simulink model "MX_Gateway"  (Simulink 9.0)
settings: Solver=FixedStepDiscrete  StartTime=0.0  StopTime=100  FixedStep=0.01  RelTol=1e-3
values from neighbouring files (symbols this model uses):
  ACTIVE = Bobcat.Parameter; CreateDefine( ACTIVE                         ,1                          ,'Define' ,'defines.h' )    [defines.m]
  ACTIVE_MODE_ONE = Bobcat.Parameter; CreateDefine( ACTIVE_MODE_ONE                ,19                         ,'Define' ,'defines.h' )    [defines.m]
  AUTHENTICATED = Bobcat.Parameter; CreateDefine( AUTHENTICATED                  ,1                          ,'Define' ,'defines.h' )    [defines.m]
  AUX_EMEA_CONTROLS = Bobcat.Parameter; CreateDefine( AUX_EMEA_CONTROLS  ,1   ,'Define' ,'defines.h' )    [enums.m]
  AUX_FLOW_HIGH = Bobcat.Parameter; CreateDefine( AUX_FLOW_HIGH           ,4  ,'Define' ,'defines.h' )    [enums.m]
  AUX_FLOW_INACTIVE = Bobcat.Parameter; CreateDefine( AUX_FLOW_INACTIVE       ,0  ,'Define' ,'defines.h' )    [enums.m]
  AUX_FLOW_LOW = Bobcat.Parameter; CreateDefine( AUX_FLOW_LOW            ,1  ,'Define' ,'defines.h' )    [enums.m]
  AUX_FLOW_MEDIUM = Bobcat.Parameter; CreateDefine( AUX_FLOW_MEDIUM         ,2  ,'Define' ,'defines.h' )    [enums.m]
  AUX_FLOW_STANDARD = Bobcat.Parameter; CreateDefine( AUX_FLOW_STANDARD       ,3  ,'Define' ,'defines.h' )    [enums.m]
  AUX_NON_FUNCTIONAL = Bobcat.Parameter; CreateDefine( AUX_NON_FUNCTIONAL ,255 ,'Define' ,'defines.h' )    [enums.m]
  BASE_ACTIVE = Bobcat.Parameter; CreateDefine( BASE_ACTIVE                    ,253                        ,'Define' ,'defines.h' )    [defines.m]
  BASE_DETENT = Bobcat.Parameter; CreateDefine( BASE_DETENT                    ,1                          ,'Define' ,'defines.h' )    [defines.m]
  BEEP_NONE = Bobcat.Parameter; CreateDefine( BEEP_NONE                      ,255                        ,'Define' ,'defines.h' )    [defines.m]
  CD_AIR_FILTER_DEBOUNCE_NORMAL = Bobcat.Parameter; CreateAliasCD( CD_AIR_FILTER_DEBOUNCE_NORMAL                        ,uint16(500)                ,'ConstantDataPointer' ,'CONSTANT_DATA' ,'AIR_FILTER_DEBOUNCE_NORMAL')    [cdata.m]    (also in hc12_cdata.m; m4_cdata.m)
  CD_AIR_FILTER_DEBOUNCE_NOT_CONNECTED = Bobcat.Parameter; CreateAliasCD( CD_AIR_FILTER_DEBOUNCE_NOT_CONNECTED                 ,uint16(3000)               ,'ConstantDataPointer' ,'CONSTANT_DATA' ,'AIR_FILTER_DEBOUNCE_NOT_CONNECTED')    [cdata.m]    (also in hc12_cdata.m; m4_cdata.m)
  CD_AIR_FILTER_DEBOUNCE_PLUGGED = Bobcat.Parameter; CreateAliasCD( CD_AIR_FILTER_DEBOUNCE_PLUGGED                       ,uint16(3000)               ,'ConstantDataPointer' ,'CONSTANT_DATA' ,'AIR_FILTER_DEBOUNCE_PLUGGED')    [cdata.m]    (also in hc12_cdata.m; m4_cdata.m)
  CD_AIR_FILTER_FUNCTION = Bobcat.Parameter; CreateAliasCD( CD_AIR_FILTER_FUNCTION                               ,uint8(255)                 ,'ConstantDataPointer' ,'CONSTANT_DATA' ,'AIR_FILTER_FUNCTION')    [cdata.m]    (also in hc12_cdata.m; m4_cdata.m)
  CD_AIR_FILTER_PLUGGED_WEIGHT = Bobcat.Parameter; CreateAliasCD( CD_AIR_FILTER_PLUGGED_WEIGHT                         ,uint8(2)                   ,'ConstantDataPointer' ,'CONSTANT_DATA' ,'AIR_FILTER_PLUGGED_WEIGHT')    [cdata.m]    (also in hc12_cdata.m; m4_cdata.m)
  CD_AIR_FILTER_UNPLUGGED_WEIGHT = Bobcat.Parameter; CreateAliasCD( CD_AIR_FILTER_UNPLUGGED_WEIGHT                       ,uint8(1)                   ,'ConstantDataPointer' ,'CONSTANT_DATA' ,'AIR_FILTER_UNPLUGGED_WEIGHT')    [cdata.m]    (also in hc12_cdata.m; m4_cdata.m)
  CD_AUTOIDLE_ARMBAR_DELAY = Bobcat.Parameter; CreateAliasCD( CD_AUTOIDLE_ARMBAR_DELAY                             ,uint8(2)                   ,'ConstantDataPointer' ,'CONSTANT_DATA' ,'AUTOIDLE_ARMBAR_DELAY')    [cdata.m]    (also in hc12_cdata.m; m4_cdata.m)
  CD_AUTOIDLE_FUNCTION = Bobcat.Parameter; CreateAliasCD( CD_AUTOIDLE_FUNCTION                                 ,uint8(255)                 ,'ConstantDataPointer' ,'CONSTANT_DATA' ,'AUTOIDLE_FUNCTION')    [cdata.m]    (also in hc12_cdata.m; m4_cdata.m)
  CD_AUTOIDLE_IDLE_DELAY = Bobcat.Parameter; CreateAliasCD( CD_AUTOIDLE_IDLE_DELAY                               ,uint8(40)                  ,'ConstantDataPointer' ,'CONSTANT_DATA' ,'AUTOIDLE_IDLE_DELAY')    [cdata.m]    (also in hc12_cdata.m; m4_cdata.m)
  CD_AUTOIDLE_LOW_IDLE_START = Bobcat.Parameter; CreateAliasCD( CD_AUTOIDLE_LOW_IDLE_START                           ,uint8(255)                 ,'ConstantDataPointer' ,'CONSTANT_DATA' ,'AUTOIDLE_LOW_IDLE_START')    [cdata.m]    (also in hc12_cdata.m; m4_cdata.m)
  CD_AUTOIDLE_PRESSURE_OFF = Bobcat.Parameter; CreateAliasCD( CD_AUTOIDLE_PRESSURE_OFF                             ,uint16(300)                ,'ConstantDataPointer' ,'CONSTANT_DATA' ,'AUTOIDLE_PRESSURE_OFF')    [cdata.m]    (also in hc12_cdata.m; m4_cdata.m)
  CD_AUTOIDLE_PRESSURE_ON = Bobcat.Parameter; CreateAliasCD( CD_AUTOIDLE_PRESSURE_ON                              ,uint16(270)                ,'ConstantDataPointer' ,'CONSTANT_DATA' ,'AUTOIDLE_PRESSURE_ON')    [cdata.m]    (also in hc12_cdata.m; m4_cdata.m)
  CD_AUTOSHIFT_FUNCTION = Bobcat.Parameter; CreateAliasCD( CD_AUTOSHIFT_FUNCTION                                ,uint8(255)                 ,'ConstantDataPointer' ,'CONSTANT_DATA' ,'AUTOSHIFT_FUNCTION')    [cdata.m]    (also in hc12_cdata.m; m4_cdata.m)
  CD_AUTOSHIFT_PHIGH1 = Bobcat.Parameter; CreateAliasCD( CD_AUTOSHIFT_PHIGH1                                  ,uint16(205)                ,'ConstantDataPointer' ,'CONSTANT_DATA' ,'AUTOSHIFT_PHIGH1')    [cdata.m]    (also in hc12_cdata.m; m4_cdata.m)
  CD_AUTOSHIFT_PHIGH2 = Bobcat.Parameter; CreateAliasCD( CD_AUTOSHIFT_PHIGH2                                  ,uint16(193)                ,'ConstantDataPointer' ,'CONSTANT_DATA' ,'AUTOSHIFT_PHIGH2')    [cdata.m]    (also in hc12_cdata.m; m4_cdata.m)
  CD_AUTOSHIFT_PHIGH_TEMP1 = Bobcat.Parameter; CreateAliasCD( CD_AUTOSHIFT_PHIGH_TEMP1                             ,int16(70)                  ,'ConstantDataPointer' ,'CONSTANT_DATA' ,'AUTOSHIFT_PHIGH_TEMP1')    [cdata.m]    (also in hc12_cdata.m; m4_cdata.m)
  CD_AUTOSHIFT_PHIGH_TEMP2 = Bobcat.Parameter; CreateAliasCD( CD_AUTOSHIFT_PHIGH_TEMP2                             ,int16(160)                 ,'ConstantDataPointer' ,'CONSTANT_DATA' ,'AUTOSHIFT_PHIGH_TEMP2')    [cdata.m]    (also in hc12_cdata.m; m4_cdata.m)
  CD_AUTOSHIFT_PLOW1 = Bobcat.Parameter; CreateAliasCD( CD_AUTOSHIFT_PLOW1                                   ,uint16(130)                ,'ConstantDataPointer' ,'CONSTANT_DATA' ,'AUTOSHIFT_PLOW1')    [cdata.m]    (also in hc12_cdata.m; m4_cdata.m)
  CD_AUTOSHIFT_PLOW2 = Bobcat.Parameter; CreateAliasCD( CD_AUTOSHIFT_PLOW2                                   ,uint16(100)                ,'ConstantDataPointer' ,'CONSTANT_DATA' ,'AUTOSHIFT_PLOW2')    [cdata.m]    (also in hc12_cdata.m; m4_cdata.m)
  CD_AUTOSHIFT_PLOW_TEMP1 = Bobcat.Parameter; CreateAliasCD( CD_AUTOSHIFT_PLOW_TEMP1                              ,int16(20)                  ,'ConstantDataPointer' ,'CONSTANT_DATA' ,'AUTOSHIFT_PLOW_TEMP1')    [cdata.m]    (also in hc12_cdata.m; m4_cdata.m)
  CD_AUTOSHIFT_PLOW_TEMP2 = Bobcat.Parameter; CreateAliasCD( CD_AUTOSHIFT_PLOW_TEMP2                              ,int16(80)                  ,'ConstantDataPointer' ,'CONSTANT_DATA' ,'AUTOSHIFT_PLOW_TEMP2')    [cdata.m]    (also in hc12_cdata.m; m4_cdata.m)
  CD_AUTOSHIFT_TRAVEL_DELAY = Bobcat.Parameter; CreateAliasCD( CD_AUTOSHIFT_TRAVEL_DELAY                            ,uint8(32)                  ,'ConstantDataPointer' ,'CONSTANT_DATA' ,'AUTOSHIFT_TRAVEL_DELAY')    [cdata.m]    (also in hc12_cdata.m; m4_cdata.m)
  CD_AUX2_CRACKPOINT_CURRENT = Bobcat.Parameter; CreateAliasCD( CD_AUX2_CRACKPOINT_CURRENT                           ,fi(0,0,16,12,'hex','0c28') ,'ConstantDataPointer' ,'CONSTANT_DATA' ,'AUX2_CRACKPOINT_CURRENT')    [cdata.m]    (also in hc12_cdata.m; m4_cdata.m)
  CD_AUX2_FLOW_LOW_FUNCTION = Bobcat.Parameter; CreateAliasCD( CD_AUX2_FLOW_LOW_FUNCTION                            ,uint8(255)                 ,'ConstantDataPointer' ,'CONSTANT_DATA' ,'AUX2_FLOW_LOW_FUNCTION')    [cdata.m]    (also in hc12_cdata.m; m4_cdata.m)
  CD_AUX2_FLOW_MED_FUNCTION = Bobcat.Parameter; CreateAliasCD( CD_AUX2_FLOW_MED_FUNCTION                            ,uint8(255)                 ,'ConstantDataPointer' ,'CONSTANT_DATA' ,'AUX2_FLOW_MED_FUNCTION')    [cdata.m]    (also in hc12_cdata.m; m4_cdata.m)
  CD_AUX2_HI_FLOW_PERCENT = Bobcat.Parameter; CreateAliasCD( CD_AUX2_HI_FLOW_PERCENT                              ,fi(0,0,16,16,'hex','ffff') ,'ConstantDataPointer' ,'CONSTANT_DATA' ,'AUX2_HI_FLOW_PERCENT')    [cdata.m]    (also in hc12_cdata.m; m4_cdata.m)
  CD_AUX2_LOW_FLOW_PERCENT = Bobcat.Parameter; CreateAliasCD( CD_AUX2_LOW_FLOW_PERCENT                             ,fi(0,0,16,16,'hex','7333') ,'ConstantDataPointer' ,'CONSTANT_DATA' ,'AUX2_LOW_FLOW_PERCENT')    [cdata.m]    (also in hc12_cdata.m; m4_cdata.m)
  CD_AUX2_MAX_FLOW_CURRENT = Bobcat.Parameter; CreateAliasCD( CD_AUX2_MAX_FLOW_CURRENT                             ,fi(0,0,16,12,'hex','14cc') ,'ConstantDataPointer' ,'CONSTANT_DATA' ,'AUX2_MAX_FLOW_CURRENT')    [cdata.m]    (also in hc12_cdata.m; m4_cdata.m)
  CD_AUX2_MED_FLOW_PERCENT = Bobcat.Parameter; CreateAliasCD( CD_AUX2_MED_FLOW_PERCENT                             ,fi(0,0,16,16,'hex','9999') ,'ConstantDataPointer' ,'CONSTANT_DATA' ,'AUX2_MED_FLOW_PERCENT')    [cdata.m]    (also in hc12_cdata.m; m4_cdata.m)
  CD_AUX_CRACKPOINT_CURRENT = Bobcat.Parameter; CreateAliasCD( CD_AUX_CRACKPOINT_CURRENT                            ,fi(0,0,16,12,'hex','0000') ,'ConstantDataPointer' ,'CONSTANT_DATA' ,'AUX_CRACKPOINT_CURRENT')    [cdata.m]    (also in hc12_cdata.m; m4_cdata.m)
  CD_AUX_DETENT_FLASH_TIME = Bobcat.Parameter; CreateAliasCD( CD_AUX_DETENT_FLASH_TIME                             ,uint16(72)                 ,'ConstantDataPointer' ,'CONSTANT_DATA' ,'AUX_DETENT_FLASH_TIME')    [cdata.m]    (also in hc12_cdata.m; m4_cdata.m)
  CD_AUX_DETENT_HOLD_TIME = Bobcat.Parameter; CreateAliasCD( CD_AUX_DETENT_HOLD_TIME                              ,uint16(100)                ,'ConstantDataPointer' ,'CONSTANT_DATA' ,'AUX_DETENT_HOLD_TIME')    [cdata.m]    (also in hc12_cdata.m; m4_cdata.m)
  CD_AUX_FULL_ON_AUX1 = Bobcat.Parameter; CreateAliasCD( CD_AUX_FULL_ON_AUX1                                  ,fi(0,0,16,12,'hex','10cd') ,'ConstantDataPointer' ,'CONSTANT_DATA' ,'AUX_FULL_ON_AUX1')    [cdata.m]    (also in hc12_cdata.m; m4_cdata.m)
  CD_AUX_FULL_ON_AUX2 = Bobcat.Parameter; CreateAliasCD( CD_AUX_FULL_ON_AUX2                                  ,fi(0,0,16,12,'hex','139d') ,'ConstantDataPointer' ,'CONSTANT_DATA' ,'AUX_FULL_ON_AUX2')    [cdata.m]    (also in hc12_cdata.m; m4_cdata.m)
  CD_AUX_FULL_ON_AUX3 = Bobcat.Parameter; CreateAliasCD( CD_AUX_FULL_ON_AUX3                                  ,fi(0,0,16,12,'hex','17d7') ,'ConstantDataPointer' ,'CONSTANT_DATA' ,'AUX_FULL_ON_AUX3')    [cdata.m]    (also in hc12_cdata.m; m4_cdata.m)
  CD_AUX_FUNCTION = Bobcat.Parameter; CreateAliasCD( CD_AUX_FUNCTION                                      ,uint8(0)                   ,'ConstantDataPointer' ,'CONSTANT_DATA' ,'AUX_FUNCTION')    [cdata.m]    (also in hc12_cdata.m; m4_cdata.m)
  CD_AUX_RATE_LIMIT_CRACKPOINT = Bobcat.Parameter; CreateAliasCD( CD_AUX_RATE_LIMIT_CRACKPOINT                         ,fi(0,0,16,12,'hex','0800') ,'ConstantDataPointer' ,'CONSTANT_DATA' ,'AUX_RATE_LIMIT_CRACKPOINT')    [cdata.m]    (also in hc12_cdata.m; m4_cdata.m)
  CD_AUX_RATE_LIMIT_CRACKPOINT_RATE = Bobcat.Parameter; CreateAliasCD( CD_AUX_RATE_LIMIT_CRACKPOINT_RATE                    ,fi(0,1,32,12,'int',102400) ,'ConstantDataPointer' ,'CONSTANT_DATA' ,'AUX_RATE_LIMIT_CRACKPOINT_RATE')    [cdata.m]    (also in hc12_cdata.m; m4_cdata.m)
  CD_AUX_RATE_LIMIT_FUNCTION = Bobcat.Parameter; CreateAliasCD( CD_AUX_RATE_LIMIT_FUNCTION                           ,uint8(255)                 ,'ConstantDataPointer' ,'CONSTANT_DATA' ,'AUX_RATE_LIMIT_FUNCTION')    [cdata.m]    (also in hc12_cdata.m; m4_cdata.m)
  CD_AUX_RATE_LIMIT_MAX_ENG_SPD = Bobcat.Parameter; CreateAliasCD( CD_AUX_RATE_LIMIT_MAX_ENG_SPD                        ,uint16(1600)               ,'ConstantDataPointer' ,'CONSTANT_DATA' ,'AUX_RATE_LIMIT_MAX_ENG_SPD')    [cdata.m]    (also in hc12_cdata.m; m4_cdata.m)
  CD_AUX_RATE_LIMIT_MIN_ENG_SPD = Bobcat.Parameter; CreateAliasCD( CD_AUX_RATE_LIMIT_MIN_ENG_SPD                        ,uint16(100)                ,'ConstantDataPointer' ,'CONSTANT_DATA' ,'AUX_RATE_LIMIT_MIN_ENG_SPD')    [cdata.m]    (also in hc12_cdata.m; m4_cdata.m)
  CD_AUX_RATE_LIMIT_NORMAL_RATE = Bobcat.Parameter; CreateAliasCD( CD_AUX_RATE_LIMIT_NORMAL_RATE                        ,fi(0,1,32,12,'int',2048)   ,'ConstantDataPointer' ,'CONSTANT_DATA' ,'AUX_RATE_LIMIT_NORMAL_RATE')    [cdata.m]    (also in hc12_cdata.m; m4_cdata.m)
  CD_AUXPOT_CAL_DEADBAND_HIGH = Bobcat.Parameter; CreateAliasCD( CD_AUXPOT_CAL_DEADBAND_HIGH                          ,uint16(574)                ,'ConstantDataPointer' ,'CONSTANT_DATA' ,'AUXPOT_CAL_DEADBAND_HIGH')    [cdata.m]    (also in hc12_cdata.m; m4_cdata.m)
  CD_AUXPOT_CAL_DEADBAND_LOW = Bobcat.Parameter; CreateAliasCD( CD_AUXPOT_CAL_DEADBAND_LOW                           ,uint16(424)                ,'ConstantDataPointer' ,'CONSTANT_DATA' ,'AUXPOT_CAL_DEADBAND_LOW')    [cdata.m]    (also in hc12_cdata.m; m4_cdata.m)
  CD_AUXPOT_CENTER_RATIO = Bobcat.Parameter; CreateAliasCD( CD_AUXPOT_CENTER_RATIO                               ,uint16(500)                ,'ConstantDataPointer' ,'CONSTANT_DATA' ,'AUXPOT_CENTER_RATIO')    [cdata.m]    (also in hc12_cdata.m; m4_cdata.m)
  CD_AUXPOT_DEADBAND = Bobcat.Parameter; CreateAliasCD( CD_AUXPOT_DEADBAND                                   ,uint8(38)                  ,'ConstantDataPointer' ,'CONSTANT_DATA' ,'AUXPOT_DEADBAND')    [cdata.m]    (also in hc12_cdata.m; m4_cdata.m)
  CD_AUXPOT_FUNCTION = Bobcat.Parameter; CreateAliasCD( CD_AUXPOT_FUNCTION                                   ,uint8(0)                   ,'ConstantDataPointer' ,'CONSTANT_DATA' ,'AUXPOT_FUNCTION')    [cdata.m]    (also in hc12_cdata.m; m4_cdata.m)
  CD_AUXPOT_RATIO_GAIN = Bobcat.Parameter; CreateAliasCD( CD_AUXPOT_RATIO_GAIN                                 ,uint16(1000)               ,'ConstantDataPointer' ,'CONSTANT_DATA' ,'AUXPOT_RATIO_GAIN')    [cdata.m]    (also in hc12_cdata.m; m4_cdata.m)
  CD_CAN_THROTTLE_FUNCTION = Bobcat.Parameter; CreateAliasCD( CD_CAN_THROTTLE_FUNCTION                             ,uint8(255)                 ,'ConstantDataPointer' ,'CONSTANT_DATA' ,'CAN_THROTTLE_FUNCTION')    [cdata.m]    (also in hc12_cdata.m; m4_cdata.m)
  CD_CAN_THROTTLE_REVERSE = Bobcat.Parameter; CreateAliasCD( CD_CAN_THROTTLE_REVERSE                              ,uint8(255)                 ,'ConstantDataPointer' ,'CONSTANT_DATA' ,'CAN_THROTTLE_REVERSE')    [cdata.m]    (also in hc12_cdata.m; m4_cdata.m)
  CD_CONSOLE_DEBOUNCE_ABSENT = Bobcat.Parameter; CreateAliasCD( CD_CONSOLE_DEBOUNCE_ABSENT                           ,uint16(12)                 ,'ConstantDataPointer' ,'CONSTANT_DATA' ,'CONSOLE_DEBOUNCE_ABSENT')    [cdata.m]    (also in hc12_cdata.m; m4_cdata.m)
  CD_CONSOLE_DEBOUNCE_OORH = Bobcat.Parameter; CreateAliasCD( CD_CONSOLE_DEBOUNCE_OORH                             ,uint16(12)                 ,'ConstantDataPointer' ,'CONSTANT_DATA' ,'CONSOLE_DEBOUNCE_OORH')    [cdata.m]    (also in hc12_cdata.m; m4_cdata.m)
  CD_CONSOLE_DEBOUNCE_OORL = Bobcat.Parameter; CreateAliasCD( CD_CONSOLE_DEBOUNCE_OORL                             ,uint16(12)                 ,'ConstantDataPointer' ,'CONSTANT_DATA' ,'CONSOLE_DEBOUNCE_OORL')    [cdata.m]    (also in hc12_cdata.m; m4_cdata.m)
  CD_CONSOLE_DEBOUNCE_PRESENT = Bobcat.Parameter; CreateAliasCD( CD_CONSOLE_DEBOUNCE_PRESENT                          ,uint16(12)                 ,'ConstantDataPointer' ,'CONSTANT_DATA' ,'CONSOLE_DEBOUNCE_PRESENT')    [cdata.m]    (also in hc12_cdata.m; m4_cdata.m)
  CD_CONSOLE_FUNCTION = Bobcat.Parameter; CreateAliasCD( CD_CONSOLE_FUNCTION                                  ,uint8(0)                   ,'ConstantDataPointer' ,'CONSTANT_DATA' ,'CONSOLE_FUNCTION')    [cdata.m]    (also in hc12_cdata.m; m4_cdata.m)
  CD_CONSOLE_OORH = Bobcat.Parameter; CreateAliasCD( CD_CONSOLE_OORH                                      ,uint16(740)                ,'ConstantDataPointer' ,'CONSTANT_DATA' ,'CONSOLE_OORH')    [cdata.m]    (also in hc12_cdata.m; m4_cdata.m)
  CD_CONSOLE_OORL = Bobcat.Parameter; CreateAliasCD( CD_CONSOLE_OORL                                      ,uint16(10)                 ,'ConstantDataPointer' ,'CONSTANT_DATA' ,'CONSOLE_OORL')    [cdata.m]    (also in hc12_cdata.m; m4_cdata.m)
  CD_CONSOLE_PRESENT_HIGH = Bobcat.Parameter; CreateAliasCD( CD_CONSOLE_PRESENT_HIGH                              ,uint16(515)                ,'ConstantDataPointer' ,'CONSTANT_DATA' ,'CONSOLE_PRESENT_HIGH')    [cdata.m]    (also in hc12_cdata.m; m4_cdata.m)
  CD_CONSOLE_PRESENT_LOW = Bobcat.Parameter; CreateAliasCD( CD_CONSOLE_PRESENT_LOW                               ,uint16(375)                ,'ConstantDataPointer' ,'CONSTANT_DATA' ,'CONSOLE_PRESENT_LOW')    [cdata.m]    (also in hc12_cdata.m; m4_cdata.m)
  CD_DELUXE_GEN5_5_FUNCTION = Bobcat.Parameter; CreateAliasCD( CD_DELUXE_GEN5_5_FUNCTION                            ,uint8(255)                 ,'ConstantDataPointer' ,'CONSTANT_DATA' ,'DELUXE_GEN5_5_FUNCTION')    [cdata.m]    (also in hc12_cdata.m; m4_cdata.m)
  CD_DELUXE_GEN5_FUNCTION = Bobcat.Parameter; CreateAliasCD( CD_DELUXE_GEN5_FUNCTION                              ,uint8(255)                 ,'ConstantDataPointer' ,'CONSTANT_DATA' ,'DELUXE_GEN5_FUNCTION')    [cdata.m]    (also in hc12_cdata.m; m4_cdata.m)
  CD_DERATE_ENGINE_SPEED_DEBOUNCE_HIGH = Bobcat.Parameter; CreateAliasCD( CD_DERATE_ENGINE_SPEED_DEBOUNCE_HIGH                 ,uint16(200)                ,'ConstantDataPointer' ,'CONSTANT_DATA' ,'DERATE_ENGINE_SPEED_DEBOUNCE_HIGH')    [cdata.m]    (also in hc12_cdata.m; m4_cdata.m)
  CD_DERATE_ENGINE_SPEED_DEBOUNCE_SHUTDOWN = Bobcat.Parameter; CreateAliasCD( CD_DERATE_ENGINE_SPEED_DEBOUNCE_SHUTDOWN             ,uint16(1000)               ,'ConstantDataPointer' ,'CONSTANT_DATA' ,'DERATE_ENGINE_SPEED_DEBOUNCE_SHUTDOWN')    [cdata.m]    (also in hc12_cdata.m; m4_cdata.m)
  CD_DERATE_ENGINE_SPEED_HIGH_OFFSET = Bobcat.Parameter; CreateAliasCD( CD_DERATE_ENGINE_SPEED_HIGH_OFFSET                   ,uint16(200)                ,'ConstantDataPointer' ,'CONSTANT_DATA' ,'DERATE_ENGINE_SPEED_HIGH_OFFSET')    [cdata.m]    (also in hc12_cdata.m; m4_cdata.m)
  CD_DERATE_METHOD = Bobcat.Parameter; CreateAliasCD( CD_DERATE_METHOD                                     ,uint8(0)                   ,'ConstantDataPointer' ,'CONSTANT_DATA' ,'DERATE_METHOD')    [cdata.m]    (also in hc12_cdata.m; m4_cdata.m)
  CD_DUMP_VALVE_FUNCTION = Bobcat.Parameter; CreateAliasCD( CD_DUMP_VALVE_FUNCTION                               ,uint8(255)                 ,'ConstantDataPointer' ,'CONSTANT_DATA' ,'DUMP_VALVE_FUNCTION')    [cdata.m]    (also in hc12_cdata.m; m4_cdata.m)
  CD_DUMP_VALVE_ON_CURRENT = Bobcat.Parameter; CreateAliasCD( CD_DUMP_VALVE_ON_CURRENT                             ,fi(0,0,16,12,'hex','ffff') ,'ConstantDataPointer' ,'CONSTANT_DATA' ,'DUMP_VALVE_ON_CURRENT')    [cdata.m]    (also in hc12_cdata.m; m4_cdata.m)
  CD_ECO_MODE_THROTTLE_REDUCTION = Bobcat.Parameter; CreateAliasCD( CD_ECO_MODE_THROTTLE_REDUCTION                       ,uint16(850)                ,'ConstantDataPointer' ,'CONSTANT_DATA' ,'ECO_MODE_THROTTLE_REDUCTION')    [cdata.m]    (also in hc12_cdata.m; m4_cdata.m)
  CD_ECT_FILTER_GAIN = Bobcat.Parameter; CreateAliasCD( CD_ECT_FILTER_GAIN                                   ,uint8(6)                   ,'ConstantDataPointer' ,'CONSTANT_DATA' ,'ECT_FILTER_GAIN')    [cdata.m]    (also in hc12_cdata.m; m4_cdata.m)
  CD_ECU_FUNCTION = Bobcat.Parameter; CreateAliasCD( CD_ECU_FUNCTION                                      ,uint8(255)                 ,'ConstantDataPointer' ,'CONSTANT_DATA' ,'ECU_FUNCTION')    [cdata.m]    (also in hc12_cdata.m; m4_cdata.m)
  CD_ECU_HOLD_DEBOUNCE_ERROR_ON = Bobcat.Parameter; CreateAliasCD( CD_ECU_HOLD_DEBOUNCE_ERROR_ON                        ,uint16(20)                 ,'ConstantDataPointer' ,'CONSTANT_DATA' ,'ECU_HOLD_DEBOUNCE_ERROR_ON')    [cdata.m]    (also in hc12_cdata.m; m4_cdata.m)
  CD_EMEA_CONTROLS_HOLD_TIME = Bobcat.Parameter; CreateAliasCD( CD_EMEA_CONTROLS_HOLD_TIME                           ,uint16(30)                 ,'ConstantDataPointer' ,'CONSTANT_DATA' ,'EMEA_CONTROLS_HOLD_TIME')    [cdata.m]    (also in hc12_cdata.m; m4_cdata.m)
  CD_EMEA_CONTROLS_OPTION_A = Bobcat.Parameter; CreateAliasCD( CD_EMEA_CONTROLS_OPTION_A                            ,uint8(255)                 ,'ConstantDataPointer' ,'CONSTANT_DATA' ,'EMEA_CONTROLS_OPTION_A')    [cdata.m]    (also in hc12_cdata.m; m4_cdata.m)
  CD_EMEA_CONTROLS_OPTION_C = Bobcat.Parameter; CreateAliasCD( CD_EMEA_CONTROLS_OPTION_C                            ,uint8(255)                 ,'ConstantDataPointer' ,'CONSTANT_DATA' ,'EMEA_CONTROLS_OPTION_C')    [cdata.m]    (also in hc12_cdata.m; m4_cdata.m)
  CD_EMEA_CONTROLS_REVERSE = Bobcat.Parameter; CreateAliasCD( CD_EMEA_CONTROLS_REVERSE                             ,uint8(255)                 ,'ConstantDataPointer' ,'CONSTANT_DATA' ,'EMEA_CONTROLS_REVERSE')    [cdata.m]    (also in hc12_cdata.m; m4_cdata.m)
  CD_EMEA_ELECTRIC_VERSION = Bobcat.Parameter; CreateAliasCD( CD_EMEA_ELECTRIC_VERSION                             ,uint8(0)                   ,'ConstantDataPointer' ,'CONSTANT_DATA' ,'EMEA_ELECTRIC_VERSION')    [cdata.m]    (also in hc12_cdata.m; m4_cdata.m)
  CD_EMEA_POT_CAL_DEADBAND_HIGH = Bobcat.Parameter; CreateAliasCD( CD_EMEA_POT_CAL_DEADBAND_HIGH                        ,uint16(574)                ,'ConstantDataPointer' ,'CONSTANT_DATA' ,'EMEA_POT_CAL_DEADBAND_HIGH')    [cdata.m]    (also in hc12_cdata.m; m4_cdata.m)
  CD_EMEA_POT_CAL_DEADBAND_LOW = Bobcat.Parameter; CreateAliasCD( CD_EMEA_POT_CAL_DEADBAND_LOW                         ,uint16(424)                ,'ConstantDataPointer' ,'CONSTANT_DATA' ,'EMEA_POT_CAL_DEADBAND_LOW')    [cdata.m]    (also in hc12_cdata.m; m4_cdata.m)
  CD_ENGINE_RPM_FACTOR = Bobcat.Parameter; CreateAliasCD( CD_ENGINE_RPM_FACTOR                                 ,uint8(35)                  ,'ConstantDataPointer' ,'CONSTANT_DATA' ,'ENGINE_RPM_FACTOR')    [cdata.m]    (also in hc12_cdata.m; m4_cdata.m)
  CD_ENGINE_SPEED_FILTER_FAST_VALUE = Bobcat.Parameter; CreateAliasCD( CD_ENGINE_SPEED_FILTER_FAST_VALUE                    ,uint8(1)                   ,'ConstantDataPointer' ,'CONSTANT_DATA' ,'ENGINE_SPEED_FILTER_FAST_VALUE')    [cdata.m]    (also in hc12_cdata.m; m4_cdata.m)
  CD_ENGINE_SPEED_FILTER_SLOW_VALUE = Bobcat.Parameter; CreateAliasCD( CD_ENGINE_SPEED_FILTER_SLOW_VALUE                    ,uint8(4)                   ,'ConstantDataPointer' ,'CONSTANT_DATA' ,'ENGINE_SPEED_FILTER_SLOW_VALUE')    [cdata.m]    (also in hc12_cdata.m; m4_cdata.m)
  CD_ENGINE_SPEED_RUNNING = Bobcat.Parameter; CreateAliasCD( CD_ENGINE_SPEED_RUNNING                              ,uint16(800)                ,'ConstantDataPointer' ,'CONSTANT_DATA' ,'ENGINE_SPEED_RUNNING')    [cdata.m]    (also in hc12_cdata.m; m4_cdata.m)
  CD_ENGINE_START_DELAY_DEBOUNCE_RPM_NO_SIGNAL = Bobcat.Parameter; CreateAliasCD( CD_ENGINE_START_DELAY_DEBOUNCE_RPM_NO_SIGNAL         ,uint16(0)                  ,'ConstantDataPointer' ,'CONSTANT_DATA' ,'ENGINE_START_DELAY_DEBOUNCE_RPM_NO_SIGNAL')    [cdata.m]    (also in hc12_cdata.m; m4_cdata.m)
  CD_ENGINE_START_DELAY_OFF_TIME = Bobcat.Parameter; CreateAliasCD( CD_ENGINE_START_DELAY_OFF_TIME                       ,uint16(0)                  ,'ConstantDataPointer' ,'CONSTANT_DATA' ,'ENGINE_START_DELAY_OFF_TIME')    [cdata.m]    (also in hc12_cdata.m; m4_cdata.m)
  CD_ENGINE_START_DELAY_RPM_NO_SIGNAL = Bobcat.Parameter; CreateAliasCD( CD_ENGINE_START_DELAY_RPM_NO_SIGNAL                  ,uint16(0)                  ,'ConstantDataPointer' ,'CONSTANT_DATA' ,'ENGINE_START_DELAY_RPM_NO_SIGNAL')    [cdata.m]    (also in hc12_cdata.m; m4_cdata.m)
  CD_EOP_DEBOUNCE_EXTREMELY_LOW = Bobcat.Parameter; CreateAliasCD( CD_EOP_DEBOUNCE_EXTREMELY_LOW                        ,uint16(50)                 ,'ConstantDataPointer' ,'CONSTANT_DATA' ,'EOP_DEBOUNCE_EXTREMELY_LOW')    [cdata.m]    (also in hc12_cdata.m; m4_cdata.m)
  CD_EOP_DEBOUNCE_NORMAL = Bobcat.Parameter; CreateAliasCD( CD_EOP_DEBOUNCE_NORMAL                               ,uint16(50)                 ,'ConstantDataPointer' ,'CONSTANT_DATA' ,'EOP_DEBOUNCE_NORMAL')    [cdata.m]    (also in hc12_cdata.m; m4_cdata.m)
  CD_EOP_DEBOUNCE_SHUTDOWN = Bobcat.Parameter; CreateAliasCD( CD_EOP_DEBOUNCE_SHUTDOWN                             ,uint16(100)                ,'ConstantDataPointer' ,'CONSTANT_DATA' ,'EOP_DEBOUNCE_SHUTDOWN')    [cdata.m]    (also in hc12_cdata.m; m4_cdata.m)
  CD_EOP_FILTER_VALUE = Bobcat.Parameter; CreateAliasCD( CD_EOP_FILTER_VALUE                                  ,uint8(3)                   ,'ConstantDataPointer' ,'CONSTANT_DATA' ,'EOP_FILTER_VALUE')    [cdata.m]    (also in hc12_cdata.m; m4_cdata.m)
  CD_EOP_FUNCTION = Bobcat.Parameter; CreateAliasCD( CD_EOP_FUNCTION                                      ,uint8(1)                   ,'ConstantDataPointer' ,'CONSTANT_DATA' ,'EOP_FUNCTION')    [cdata.m]    (also in hc12_cdata.m; m4_cdata.m)
  CD_EOP_SWITCH_GAUGE_VALUE = Bobcat.Parameter; CreateAliasCD( CD_EOP_SWITCH_GAUGE_VALUE                            ,uint16(125)                ,'ConstantDataPointer' ,'CONSTANT_DATA' ,'EOP_SWITCH_GAUGE_VALUE')    [cdata.m]    (also in hc12_cdata.m; m4_cdata.m)
  CD_EOP_SWITCH_LOW = Bobcat.Parameter; CreateAliasCD( CD_EOP_SWITCH_LOW                                    ,uint16(50)                 ,'ConstantDataPointer' ,'CONSTANT_DATA' ,'EOP_SWITCH_LOW')    [cdata.m]    (also in hc12_cdata.m; m4_cdata.m)
  CD_EOP_SWITCH_PRESSURE_VALUE = Bobcat.Parameter; CreateAliasCD( CD_EOP_SWITCH_PRESSURE_VALUE                         ,uint16(40)                 ,'ConstantDataPointer' ,'CONSTANT_DATA' ,'EOP_SWITCH_PRESSURE_VALUE')    [cdata.m]    (also in hc12_cdata.m; m4_cdata.m)
  CD_EOT_DEBOUNCE_EXTREMELY_LOW = Bobcat.Parameter; CreateAliasCD( CD_EOT_DEBOUNCE_EXTREMELY_LOW                        ,uint16(2)                  ,'ConstantDataPointer' ,'CONSTANT_DATA' ,'EOT_DEBOUNCE_EXTREMELY_LOW')    [cdata.m]    (also in hc12_cdata.m; m4_cdata.m)
  CD_EOT_DEBOUNCE_LOW = Bobcat.Parameter; CreateAliasCD( CD_EOT_DEBOUNCE_LOW                                  ,uint16(2)                  ,'ConstantDataPointer' ,'CONSTANT_DATA' ,'EOT_DEBOUNCE_LOW')    [cdata.m]    (also in hc12_cdata.m; m4_cdata.m)
  CD_EOT_DEBOUNCE_NORMAL = Bobcat.Parameter; CreateAliasCD( CD_EOT_DEBOUNCE_NORMAL                               ,uint16(2)                  ,'ConstantDataPointer' ,'CONSTANT_DATA' ,'EOT_DEBOUNCE_NORMAL')    [cdata.m]    (also in hc12_cdata.m; m4_cdata.m)
  CD_EOT_EXTREMELY_LOW = Bobcat.Parameter; CreateAliasCD( CD_EOT_EXTREMELY_LOW                                 ,uint16(81)                 ,'ConstantDataPointer' ,'CONSTANT_DATA' ,'EOT_EXTREMELY_LOW')    [cdata.m]    (also in hc12_cdata.m; m4_cdata.m)
  CD_EOT_FUNCTION = Bobcat.Parameter; CreateAliasCD( CD_EOT_FUNCTION                                      ,uint8(255)                 ,'ConstantDataPointer' ,'CONSTANT_DATA' ,'EOT_FUNCTION')    [cdata.m]    (also in hc12_cdata.m; m4_cdata.m)
  CD_EOT_LOW = Bobcat.Parameter; CreateAliasCD( CD_EOT_LOW                                           ,uint16(90)                 ,'ConstantDataPointer' ,'CONSTANT_DATA' ,'EOT_LOW')    [cdata.m]    (also in hc12_cdata.m; m4_cdata.m)
  CD_EOT_TEMPERATURE_HYSTERESIS = Bobcat.Parameter; CreateAliasCD( CD_EOT_TEMPERATURE_HYSTERESIS                        ,uint16(2)                  ,'ConstantDataPointer' ,'CONSTANT_DATA' ,'EOT_TEMPERATURE_HYSTERESIS')    [cdata.m]    (also in hc12_cdata.m; m4_cdata.m)
  CD_FAST_TRAVEL_FUNCTION = Bobcat.Parameter; CreateAliasCD( CD_FAST_TRAVEL_FUNCTION                              ,uint8(255)                 ,'ConstantDataPointer' ,'CONSTANT_DATA' ,'FAST_TRAVEL_FUNCTION')    [cdata.m]    (also in hc12_cdata.m; m4_cdata.m)
  CD_FAST_TRAVEL_THROTTLE_DELAY = Bobcat.Parameter; CreateAliasCD( CD_FAST_TRAVEL_THROTTLE_DELAY                        ,uint16(10)                 ,'ConstantDataPointer' ,'CONSTANT_DATA' ,'FAST_TRAVEL_THROTTLE_DELAY')    [cdata.m]    (also in hc12_cdata.m; m4_cdata.m)
  CD_FAST_TRAVEL_THROTTLE_LIMIT = Bobcat.Parameter; CreateAliasCD( CD_FAST_TRAVEL_THROTTLE_LIMIT                        ,uint16(1000)               ,'ConstantDataPointer' ,'CONSTANT_DATA' ,'FAST_TRAVEL_THROTTLE_LIMIT')    [cdata.m]    (also in hc12_cdata.m; m4_cdata.m)
  CD_FLYWHEEL_TEETH = Bobcat.Parameter; CreateAliasCD( CD_FLYWHEEL_TEETH                                    ,uint8(83)                  ,'ConstantDataPointer' ,'CONSTANT_DATA' ,'FLYWHEEL_TEETH')    [cdata.m]    (also in hc12_cdata.m; m4_cdata.m)
  CD_FUEL_LEVEL_FILTER_FAST_VALUE = Bobcat.Parameter; CreateAliasCD( CD_FUEL_LEVEL_FILTER_FAST_VALUE                      ,uint8(5)                   ,'ConstantDataPointer' ,'CONSTANT_DATA' ,'FUEL_LEVEL_FILTER_FAST_VALUE')    [cdata.m]    (also in hc12_cdata.m; m4_cdata.m)
  CD_FUEL_LEVEL_FILTER_SLOW_VALUE = Bobcat.Parameter; CreateAliasCD( CD_FUEL_LEVEL_FILTER_SLOW_VALUE                      ,uint8(10)                  ,'ConstantDataPointer' ,'CONSTANT_DATA' ,'FUEL_LEVEL_FILTER_SLOW_VALUE')    [cdata.m]    (also in hc12_cdata.m; m4_cdata.m)
  CD_FUEL_PRIMARY_FEEDBACK_FUNCTION = Bobcat.Parameter; CreateAliasCD( CD_FUEL_PRIMARY_FEEDBACK_FUNCTION                    ,uint8(0)                   ,'ConstantDataPointer' ,'CONSTANT_DATA' ,'FUEL_PRIMARY_FEEDBACK_FUNCTION')    [cdata.m]    (also in hc12_cdata.m; m4_cdata.m)
  ... (+246 more)
undefined symbols (not defined in the file or in its folder): sigbuilder_block
undefined symbols (not defined in the file): CD_FUEL_RESOLUTION_TIMER
libraries: simulink x186, MX_Library x107
(part 13 of 48)

== system MX_Gateway/MX_Gtwy_Control/Inputs/EMEA_Left_Control ==
  state_System : Inport
  ain_LeftPot : Inport  Port=2
  ain_SensorSupply2 : Inport  Port=3
  state_JoystickEnable : Inport  Port=4
  state_Console : Inport  Port=5
  state_Engine : Inport  Port=6
  check_LeftNeutral : Inport  Port=7
  state_SensorSupply2 : Inport  Port=8
  Compare To Constant : simulink/Logic and Bit Operations/Compare To Constant  relop===  const=SYS_NORMAL  OutDataTypeStr=boolean
  CompareToConstant1 : simulink/Logic and Bit Operations/Compare To Constant  relop=~=  const=NON_FUNCTIONAL  OutDataTypeStr=boolean  ZeroCross=off
  Constant3 : Constant  Value=CD_LEFT_POT_FUNCTION
  Data Type Conversion3 : DataTypeConversion  OutDataTypeStr=uint16
  Logical Operator : Logic  AllPortsSameDT=off  OutDataTypeStr=boolean
  Normal_Pot : Subsystem  RTWFileName=mod_Inputs
      settings: RTWSystemCode=Nonreusable function  RTWFileNameOpts=User specified
  Saturation : Saturate  UpperLimit=1000  LowerLimit=0
      settings: AttributesFormatString=Upper Limit = %<UpperLimit>\nLower Limit = %<LowerLimit>
  state_LeftPot : Outport
  position_LeftPot : Outport  Port=2
  rm_LeftPot : Outport  Port=3
  wires:
  ain_LeftPot/1 -> Normal_Pot/1(ain_LeftPot)
  state_Console/1 -> Normal_Pot/3(state_Console)
  state_Engine/1 -> Normal_Pot/4(state_Engine)
  state_JoystickEnable/1 -> Normal_Pot/5(state_JoystickEnable)
  ain_SensorSupply2/1 -> Normal_Pot/2(ain_SensorSupply2)
  Saturation/1 -> position_LeftPot/1
  state_SensorSupply2/1 -> Normal_Pot/6(state_SensorSupply2)
  Normal_Pot/1(state_LeftPot) -> state_LeftPot/1
  Normal_Pot/2(position_LeftPot) -> Data Type Conversion3/1
  Normal_Pot/3(rm_LeftPot) -> rm_LeftPot/1
  state_System/1 -> Compare To Constant/1
  Compare To Constant/1 -> Logical Operator/1
  Logical Operator/1 -> Normal_Pot/Enable
  check_LeftNeutral/1 -> Normal_Pot/7(check_LeftNeutral)
  Data Type Conversion3/1 -> Saturation/1
  Constant3/1 -> CompareToConstant1/1
  CompareToConstant1/1 -> Logical Operator/2

== system MX_Gateway/MX_Gtwy_Control/Inputs/EMEA_Right_Control ==
  state_AuxEnable : Inport
  aux_Flow : Inport  Port=2
  state_Engine : Inport  Port=3
  check_RightNeutral : Inport  Port=4
  aux_Inhibit : Inport  Port=5
  mode_RightControl : Inport  Port=6
  ain_RightPot : Inport  Port=7
  ain_SensorSupply2 : Inport  Port=8
  state_System : Inport  Port=9
  aux_DetentHold : Inport  Port=10
  aux_DebounceButton : Inport  Port=11
  state_Console : Inport  Port=12
  state_SensorSupply2 : Inport  Port=13
  Compare To Constant : simulink/Logic and Bit Operations/Compare To Constant  relop===  const=SYS_NORMAL  OutDataTypeStr=boolean
  CompareToConstant1 : simulink/Logic and Bit Operations/Compare To Constant  relop=~=  const=NON_FUNCTIONAL  OutDataTypeStr=boolean  ZeroCross=off
  Constant2 : Constant  Value=CD_RIGHT_POT_FUNCTION
  Logical Operator : Logic  AllPortsSameDT=off  OutDataTypeStr=boolean
  Right Pot : Subsystem  RTWFileName=mod_Inputs
      settings: RTWSystemCode=Nonreusable function  RTWFileNameOpts=User specified
  state_RightPot : Outport
  position_RightPot : Outport  Port=2
  rm_RightPot : Outport  Port=3
  wires:
  state_SensorSupply2/1 -> Right Pot/9(state_SensorSupply2)
  aux_DebounceButton/1 -> Right Pot/7(aux_DebounceButton)
  state_AuxEnable/1 -> Right Pot/1(state_AuxEnable)
  ain_RightPot/1 -> Right Pot/4(ain_RightPot)
  state_Console/1 -> Right Pot/8(state_Console)
  aux_DetentHold/1 -> Right Pot/6(aux_DetentHold)
  ain_SensorSupply2/1 -> Right Pot/5(ain_SensorSupply2)
  state_Engine/1 -> Right Pot/3(state_Engine)
  aux_Flow/1 -> Right Pot/2(aux_Flow)
  Right Pot/3(rm_RightPot) -> rm_RightPot/1
  Right Pot/2(position_RightPot) -> position_RightPot/1
  Right Pot/1(state_RightPot) -> state_RightPot/1
  state_System/1 -> Compare To Constant/1
  Compare To Constant/1 -> Logical Operator/1
  Logical Operator/1 -> Right Pot/Enable
  check_RightNeutral/1 -> Right Pot/10(check_RightNeutral)
  aux_Inhibit/1 -> Right Pot/11(aux_Inhibit)
  mode_RightControl/1 -> Right Pot/12(mode_RightControl)
  Constant2/1 -> CompareToConstant1/1
  CompareToConstant1/1 -> Logical Operator/2

== system MX_Gateway/MX_Gtwy_Control/Inputs/EOP ==
  adin_EOP : Inport  OutDataTypeStr=uint16
  ain_SensorSupply1 : Inport  Port=2  OutDataTypeStr=uint16
  ain_SensorSupply2 : Inport  Port=3  OutDataTypeStr=uint16
  state_System : Inport  Port=4
  ecu_EOP : Inport  Port=5
  state_CommECU : Inport  Port=6
  state_SensorSupply1 : Inport  Port=7  OutDataTypeStr=uint8
  state_SensorSupply2 : Inport  Port=8  OutDataTypeStr=uint8
  state_Engine : Inport  Port=9  OutDataTypeStr=uint8
  flt_EngineSpeed : Inport  Port=10  OutDataTypeStr=uint16
  Compare to Sender : simulink/Logic and Bit Operations/Compare To Constant  relop=~=  const=SWITCH_INPUT  OutDataTypeStr=boolean  ZeroCross=off
  Compare to Switch : simulink/Logic and Bit Operations/Compare To Constant  relop===  const=SWITCH_INPUT  OutDataTypeStr=boolean  ZeroCross=off
  Constant2 : Constant  Value=CD_EOP_FUNCTION  OutDataTypeStr=uint8
  SensorInput : Subsystem  TreatAsAtomicUnit=on
  SwitchInput : Subsystem  TreatAsAtomicUnit=on
  sel_State : Switch  Criteria=u2 ~= 0  ZeroCross=off
  sel_State1 : Switch  Criteria=u2 ~= 0  ZeroCross=off
  sel_State2 : Switch  Criteria=u2 ~= 0  ZeroCross=off
  state_EOP : Outport  OutDataTypeStr=uint8
  flt_EOP : Outport  Port=2  OutDataTypeStr=uint16
  gau_EOP : Outport  Port=3
  outputSelect_EOP : Outport  Port=4
  rm_EOP : Outport  Port=5
  level_ShutdownEOP : Outport  Port=6
  level_WarningEOP : Outport  Port=7
  wires:
  SensorInput/4(rm_EOP) -> rm_EOP/1  "rm_EOP"
  Compare to Sender/1 -> SensorInput/Enable
  [adin_EOP] -> SwitchInput/1(adin_EOP)  "adin_EOP"
  sel_State/1 -> state_EOP/1  "state_EOP"
  SwitchInput/1(state_EOPSw) -> sel_State/1  "state_EOPSw"
  SensorInput/1(state_EOPSr) -> sel_State/3  "state_EOPSr"
  adin_EOP/1 -> [adin_EOP]
  Constant2/1 -> Compare to Switch/1, Compare to Sender/1  "EOP_Function"
  Compare to Switch/1 -> sel_State2/2, sel_State1/2, sel_State/2, outputSelect_EOP/1, SwitchInput/Enable  "outputSelectEOP"
  state_Engine/1 -> [state_Engine]
  [state_Engine] -> SwitchInput/2(state_Engine)  "state_Engine"
  SensorInput/5(level_WarningEOP) -> level_WarningEOP/1  "level_WarningEOP"
  SensorInput/6(level_ShutdownEOP) -> level_ShutdownEOP/1  "level_ShutdownEOP"
  [adin_EOP] -> SensorInput/1(adin_EOP)  "adin_EOP"
  ain_SensorSupply1/1 -> SensorInput/2(ain_SensorSupply1)  "ain_SensorSupply1"
  ain_SensorSupply2/1 -> SensorInput/3(ain_SensorSupply2)  "ain_SensorSupply2"
  state_SensorSupply1/1 -> SensorInput/4(state_SensorSupply1)  "state_SensorSupply1"
  state_SensorSupply2/1 -> SensorInput/5(state_SensorSupply2)  "state_SensorSupply2"
  [state_Engine] -> SensorInput/6(state_Engine)  "state_Engine"
  flt_EngineSpeed/1 -> SensorInput/7(flt_EngineSpeed)  "flt_EngineSpeed"
  state_System/1 -> SensorInput/8(state_System)  "state_System"
  ecu_EOP/1 -> SensorInput/9(ecu_EOP)  "ecu_EOP"
  state_CommECU/1 -> SensorInput/10(state_CommECU)  "state_CommECU"
  sel_State1/1 -> flt_EOP/1
  sel_State2/1 -> gau_EOP/1
  SwitchInput/2(flt_EOP) -> sel_State1/1
  SensorInput/2(flt_EOP) -> sel_State1/3  "flt_EOP"
  SwitchInput/3(gau_EOP) -> sel_State2/1
  SensorInput/3(gau_EOP) -> sel_State2/3  "gau_EOP"

== system MX_Gateway/MX_Gtwy_Control/Inputs/Eco_Mode ==
  button_EcoMode : Inport
  flag_InitEERead : Inport  Port=2
  dlx_G55_EcoMode : Inport  Port=3
  Eco_Mode : Stateflow chart  TreatAsAtomicUnit=on
  state_EcoMode : Outport
  wires:
  button_EcoMode/1 -> Eco_Mode/1(button_EcoMode)
  Eco_Mode/1(state_EcoMode) -> state_EcoMode/1
  flag_InitEERead/1 -> Eco_Mode/2(flag_InitEERead)
  dlx_G55_EcoMode/1 -> Eco_Mode/3(dlx_G55_EcoMode)

== system MX_Gateway/MX_Gtwy_Control/Inputs/EngineSpeed ==
  freq_EngineSpeed : Inport
  adin_EOP : Inport  Port=2
  state_System : Inport  Port=3
  ecu_EngineSpeed : Inport  Port=4
  state_CommECU : Inport  Port=5
  flt_EOP : Inport  Port=6
  state_EOP : Inport  Port=7
  state_SensorSupply2 : Inport  Port=8
  Compare To Constant : simulink/Logic and Bit Operations/Compare To Constant  relop===  const=NON_FUNCTIONAL  OutDataTypeStr=boolean  ZeroCross=off
  Compare To Constant1 : simulink/Logic and Bit Operations/Compare To Constant  relop===  const=NON_FUNCTIONAL  OutDataTypeStr=boolean  ZeroCross=off
  Constant : Constant  Value=CD_ECU_FUNCTION  OutDataTypeStr=uint8
  Constant1 : Constant  Value=CD_ECU_FUNCTION  OutDataTypeStr=uint8
  Data Type Conversion1 : DataTypeConversion
  Data Type Conversion2 : DataTypeConversion
  EngineSpeed : Stateflow chart  TreatAsAtomicUnit=on
  Gain : Stateflow chart  TreatAsAtomicUnit=on
  Switch : Switch  Criteria=u2 ~= 0
  calculate_engine_speed : Subsystem
  flt_EngineSpeed : Outport  OutDataTypeStr=uint16
  state_EngineSpeed : Outport  Port=2  OutDataTypeStr=uint8
  calc_EngineSpeed : Outport  Port=3  OutDataTypeStr=uint16
  gain_EngineSpeed : Outport  Port=4
  wires:
  adin_EOP/1 -> EngineSpeed/5(adin_EOP)  "adin_EOP"
  state_System/1 -> EngineSpeed/4(state_System)  "state_System"
  state_EOP/1 -> EngineSpeed/2(state_EOP)  "state_EOP"
  EngineSpeed/1(state_EngineSpeed) -> state_EngineSpeed/1  "state_EngineSpeed"
  flt_EOP/1 -> EngineSpeed/3(flt_EOP)  "flt_EOP"
  state_CommECU/1 -> EngineSpeed/6(state_CommECU)  "state_CommECU"
  freq_EngineSpeed/1 -> calculate_engine_speed/1(freq_EngineSpeed)  "freq_EngineSpeed"
  Data Type Conversion1/1 -> EngineSpeed/1(flt_EngineSpeed_in)  "flt_EngineSpeed"
  state_SensorSupply2/1 -> EngineSpeed/7(state_SensorSupply2)  "state_SensorSupply2"
  Gain/2(flt_EngineSpeed) -> Data Type Conversion1/1
  Data Type Conversion2/1 -> Gain/1(convEngineSpeed)  "convEngineSpeed"
  Gain/1(gain_EngineSpeed) -> gain_EngineSpeed/1  "gain_EngineSpeed"
  calculate_engine_speed/1(calc_EngineSpeed) -> calc_EngineSpeed/1, Switch/1  "calc_EngineSpeed"
  Switch/1 -> Data Type Conversion2/1
  Constant/1 -> Compare To Constant/1
  Compare To Constant/1 -> calculate_engine_speed/Enable
  Constant1/1 -> Compare To Constant1/1
  Compare To Constant1/1 -> Switch/2
  ecu_EngineSpeed/1 -> Switch/3  "ecu_EngineSpeed"
  EngineSpeed/2(flt_EngineSpeed_out) -> flt_EngineSpeed/1  "flt_EngineSpeed"

== system MX_Gateway/MX_Gtwy_Control/Inputs/Engine_Oil_Temperature ==
  ecu_EOT : Inport  OutDataTypeStr=uint16
  state_System : Inport  Port=2
  state_ecuECT : Inport  Port=3
  state_Engine : Inport  Port=4
  ErrorDetection : Stateflow chart  TreatAsAtomicUnit=on
  state_EOT : Outport
  wires:
  state_System/1 -> ErrorDetection/2(state_System)  "state_System"
  state_Engine/1 -> ErrorDetection/3(state_Engine)  "state_Engine"
  ecu_EOT/1 -> ErrorDetection/1(ecu_EOT)  "ecu_EOT"
  ErrorDetection/1(state_EOT) -> state_EOT/1  "state_EOT"
  state_ecuECT/1 -> ErrorDetection/4(state_ecuECT)  "state_ecuECT"

== system MX_Gateway/MX_Gtwy_Control/Inputs/Engine_Start ==
  din_EngineStart : Inport
  can_ST_EngineStart : Inport  Port=2
  can_ACD_EngineStart : Inport  Port=3
  state_ST_CAN : Inport  Port=4
  state_ACD_CAN : Inport  Port=5
  din_RemoteKey : Inport  Port=6
  state_MachineLock : Inport  Port=7
  state_Engine : Inport  Port=8
  flt_EngineSpeed : Inport  Port=9
  Engine_Start : Stateflow chart  TreatAsAtomicUnit=on
  state_EngineStart : Outport
  wires:
  Engine_Start/1(state_EngineStart) -> state_EngineStart/1  "state_EngineStart"
  din_EngineStart/1 -> Engine_Start/1(din_EngineStart)  "din_EngineStart"
  can_ST_EngineStart/1 -> Engine_Start/2(can_ST_EngineStart)  "can_ST_EngineStart"
  state_ST_CAN/1 -> Engine_Start/4(state_ST_CAN03)  "state_ST_CAN03"
  state_MachineLock/1 -> Engine_Start/6(state_MachineLock)  "state_MachineLock"
  din_RemoteKey/1 -> Engine_Start/7(din_RemoteKey)  "din_RemoteKey"
  can_ACD_EngineStart/1 -> Engine_Start/3(can_ACD_EngineStart)
  state_ACD_CAN/1 -> Engine_Start/5(state_ACD_CAN03)
  state_Engine/1 -> Engine_Start/8(state_Engine)
  flt_EngineSpeed/1 -> Engine_Start/9(flt_EngineSpeed)

== system MX_Gateway/MX_Gtwy_Control/Inputs/FuelLevel ==
  ain_FuelLevel : Inport  OutDataTypeStr=uint16
  ain_SwitchedPower : Inport  Port=2  OutDataTypeStr=uint16
  state_Console : Inport  Port=3
  state_Engine : Inport  Port=4
  Data Type Conversion1 : DataTypeConversion  OutDataTypeStr=uint16
  Data Type Conversion2 : DataTypeConversion
  Data Type Conversion4 : DataTypeConversion
  Data Type Conversion5 : DataTypeConversion
  Fuel Gauge Table1 : Lookup  InputValues=MXGTW_FUELSND  Table=MXGTW_FUELGAUGE  LookUpMeth=Use Input Nearest
      settings: AttributesFormatString=input = %<InputValues>\noutput = %<OutputValues>\nlookup = %<LookUpMeth>\nrounding = %<RndMeth>
  Fuel_Level : Stateflow chart  TreatAsAtomicUnit=on
  Gain : Stateflow chart  TreatAsAtomicUnit=on
  LP_Filter_Adj_Gain : MX_Library/LP_Filter_Adj_Gain
  LP_Filter_Adj_Gain1 : MX_Library/LP_Filter_Adj_Gain
  Multiport Switch : MultiPortSwitch  OutDataTypeStr=uint16
  Ratiometric_Converter : MX_Library/Ratiometric_Converter  multiplier=1000
  Remove_OOR_Levels : MX_Library/Remove_OOR_Levels  OORL_LEVEL=CD_FUEL_LEVEL_OORL  OORH_LEVEL=CD_FUEL_LEVEL_OORH
  Truth_Table : Truth Table  TreatAsAtomicUnit=on
  filter_level : Constant  Value=3  OutDataTypeStr=uint8
  max : Constant  Value=250  OutDataTypeStr=uint16
  min : Constant  Value=0  OutDataTypeStr=uint16  SampleTime=srate100
  state_FuelLevel : Outport
  gau_FuelLevel : Outport  Port=2
  rm_FuelLevel : Outport  Port=3
  wires:
  Fuel_Level/1(state_FuelLevel) -> state_FuelLevel/1, Truth_Table/1(state)  "state_FuelLevel"
  ain_SwitchedPower/1 -> Ratiometric_Converter/2  "ain_SwitchedPower"
  LP_Filter_Adj_Gain/1 -> Data Type Conversion1/1
  Gain/1(Gain) -> LP_Filter_Adj_Gain/1  "Gain"
  ain_FuelLevel/1 -> Ratiometric_Converter/1  "ain_FuelLevel"
  Ratiometric_Converter/1 -> Data Type Conversion5/1, Remove_OOR_Levels/1  "ratiometric"
  Data Type Conversion2/1 -> LP_Filter_Adj_Gain/2  "ratiometric"
  Multiport Switch/1 -> gau_FuelLevel/1
  min/1 -> Multiport Switch/2
  max/1 -> Multiport Switch/4
  LP_Filter_Adj_Gain1/1 -> Data Type Conversion4/1
  filter_level/1 -> LP_Filter_Adj_Gain1/1
  Data Type Conversion5/1 -> LP_Filter_Adj_Gain1/2
  state_Console/1 -> Gain/1(state_Console), Fuel_Level/2(state_Console)
  state_Engine/1 -> Fuel_Level/1(state_Engine)
  Truth_Table/1(gauge) -> Multiport Switch/1
  [fltValue] -> Multiport Switch/3
  Data Type Conversion4/1 -> rm_FuelLevel/1, Fuel_Level/4(rawValue)
  Fuel Gauge Table1/1 -> Data Type Conversion2/1  "fltValue"
  Data Type Conversion1/1 -> Fuel_Level/3(fltValue), [fltValue]
  Remove_OOR_Levels/1 -> Fuel Gauge Table1/1

== system MX_Gateway/MX_Gtwy_Control/Inputs/Fuel_Temperature ==
  can_FuelTemp : Inport
  state_System : Inport  Port=2
  state_CommECU : Inport  Port=3
  state_Engine : Inport  Port=4
  ErrorDetection : Stateflow chart  TreatAsAtomicUnit=on
  state_FuelTemp : Outport
  wires:
  state_System/1 -> ErrorDetection/2(state_System)  "state_System"
  state_Engine/1 -> ErrorDetection/3(state_Engine)  "state_Engine"
  can_FuelTemp/1 -> ErrorDetection/1(can_FuelTemp)  "can_FuelTemp"
  state_CommECU/1 -> ErrorDetection/4(state_CommECU)  "state_CommECU"
  ErrorDetection/1(state_FuelTemp) -> state_FuelTemp/1  "state_FuelTemp"

== system MX_Gateway/MX_Gtwy_Control/Inputs/HCFilter1 ==
  din_HCFilter1 : Inport  OutDataTypeStr=uint8
  state_Engine : Inport  Port=2  OutDataTypeStr=uint8
  state_HOT : Inport  Port=3  OutDataTypeStr=uint8
  Hyd1_Filter_State : Stateflow chart  TreatAsAtomicUnit=on
  state_HCFilter1 : Outport  OutDataTypeStr=uint8
  wires:
  din_HCFilter1/1 -> Hyd1_Filter_State/1(din_HCFilter1)  "din_HCFilter1"
  state_Engine/1 -> Hyd1_Filter_State/2(state_Engine)  "state_Engine"
  Hyd1_Filter_State/1(state_HCFilter1) -> state_HCFilter1/1  "state_HCFilter1"
  state_HOT/1 -> Hyd1_Filter_State/3(state_HOT)  "state_HOT"

== system MX_Gateway/MX_Gtwy_Control/Inputs/HydOilTemp ==
  adin_HOT : Inport  OutDataTypeStr=uint16
  ain_SensorSupply1 : Inport  Port=2  OutDataTypeStr=uint16
  state_System : Inport  Port=3
  state_SensorSupply1 : Inport  Port=4  OutDataTypeStr=uint8
  state_Engine : Inport  Port=5  OutDataTypeStr=uint8
  Compare To SENDER : simulink/Logic and Bit Operations/Compare To Constant  relop===  const=SENDER  OutDataTypeStr=boolean  ZeroCross=off
  Compare To SWITCH : simulink/Logic and Bit Operations/Compare To Constant  relop===  const=SWITCH_INPUT  OutDataTypeStr=boolean  ZeroCross=off
  Constant2 : Constant  Value=CD_HOT_FUNCTION  OutDataTypeStr=uint8
  SenderState : Subsystem  TreatAsAtomicUnit=on
  Switch : Switch  Criteria=u2 > Threshold  ZeroCross=off
      settings: AttributesFormatString=<threshold=%<Threshold>>
  SwitchState : Subsystem  TreatAsAtomicUnit=on
  state_HOT : Outport  OutDataTypeStr=uint8
  flt_HOT : Outport  Port=2  OutDataTypeStr=uint16
  gau_HOT : Outport  Port=3  OutDataTypeStr=uint16
  outputSelect_HOT : Outport  Port=4
  rm_HOT : Outport  Port=5  OutDataTypeStr=uint16
  wires:
  state_SensorSupply1/1 -> SenderState/3(state_SensorSupply1)  "state_SensorSupply1"
  ain_SensorSupply1/1 -> SenderState/2(ain_SensorSupply1)  "ain_SensorSupply1"
  [adin_HOT] -> SenderState/1(adin_HOT)  "adin_HOT"
  [adin_HOT] -> SwitchState/1(adin_HOT)  "adin_HOT"
  Switch/1 -> state_HOT/1  "state_HOT"
  SwitchState/1(state_HOT) -> Switch/1
  SenderState/1(state_HOT) -> Switch/3
  SenderState/3(gau_HOT) -> gau_HOT/1  "gau_HOT"
  SenderState/2(flt_HOT) -> flt_HOT/1  "flt_HOT"
  adin_HOT/1 -> [adin_HOT]  "adin_HOT"
  Constant2/1 -> Compare To SWITCH/1, Compare To SENDER/1  "Function"
  Compare To SENDER/1 -> SenderState/Enable
  Compare To SWITCH/1 -> Switch/2, SwitchState/Enable, outputSelect_HOT/1  "outputSelect_HOT"
  [state_System] -> SenderState/4(state_System)  "state_System"
  state_System/1 -> [state_System]
  [state_System] -> SwitchState/2(state_System)  "state_System"
  SenderState/4(rm_HOT) -> rm_HOT/1  "rm_HOT"
  state_Engine/1 -> SenderState/5(state_Engine)  "state_Engine"

== system MX_Gateway/MX_Gtwy_Control/Inputs/Joystick_Pots ==
  BJM2_Right : Inport
  BJM2_Left : Inport  Port=2
  Bitwise Operator1 : simulink/Logic and Bit Operations/Bitwise Operator  logicop=OR  UseBitMask=off  NumInputPorts=2  BitMask=bin2dec('11011001')  BitMaskRealWorld=Stored Integer
  Bitwise Operator2 : simulink/Logic and Bit Operations/Bitwise Operator  logicop=OR  UseBitMask=off  NumInputPorts=2  BitMask=bin2dec('11011001')  BitMaskRealWorld=Stored Integer
  Data Type Conversion{1..2,4,6} (4 alike) : DataTypeConversion  OutDataTypeStr=uint16
  Demux1 : Demux  Outputs=8
  Demux2 : Demux  Outputs=8
  Left : Subsystem
  Right : Subsystem
  Shift Arithmetic1 : ArithShift  BitShiftNumber=8  BitShiftDirection=Left
  Shift Arithmetic2 : ArithShift  BitShiftNumber=8  BitShiftDirection=Left
  Terminator{1..10,18} (11 alike) : Terminator
  cmd_PotRight : Outport
  can_TriggerRight : Outport  Port=2
  cmd_PotLeft : Outport  Port=3
  wires:
  BJM2_Left/1 -> Demux1/1
  Demux1/1 -> Data Type Conversion6/1
  Demux1/2 -> Data Type Conversion4/1
  Data Type Conversion4/1 -> Shift Arithmetic1/1
  Shift Arithmetic1/1 -> Bitwise Operator2/2
  Bitwise Operator2/1 -> Left/1(Pot_Raw)
  Demux1/3 -> Terminator6/1
  Demux1/4 -> Terminator7/1
  Demux1/6 -> Terminator3/1
  Demux1/7 -> Terminator4/1
  Demux1/8 -> Terminator5/1
  Data Type Conversion6/1 -> Bitwise Operator2/1
  BJM2_Right/1 -> Demux2/1
  Demux2/5 -> Right/2(Buttons_5_8)
  Demux2/1 -> Data Type Conversion2/1
  Demux2/2 -> Data Type Conversion1/1
  Data Type Conversion1/1 -> Shift Arithmetic2/1
  Shift Arithmetic2/1 -> Bitwise Operator1/2
  Bitwise Operator1/1 -> Right/1(Pot_Raw)
  Demux2/3 -> Terminator9/1
  Demux2/4 -> Terminator10/1
  Demux2/6 -> Terminator1/1
  Demux2/7 -> Terminator2/1
  Demux2/8 -> Terminator8/1
  Data Type Conversion2/1 -> Bitwise Operator1/1
  Right/1(Pot_Cmd) -> cmd_PotRight/1
  Right/2(Trigger) -> can_TriggerRight/1
  Left/1(Pot_Cmd) -> cmd_PotLeft/1  "Pot_Cmd"
  Demux1/5 -> Terminator18/1
